simulink model "TosAeb"  (R2021a)
settings: Solver=FixedStepDiscrete  StartTime=0.0  StopTime=inf  FixedStep=0.01  RelTol=1e-3
undefined symbols (not defined in the file or in its folder): ACCModSts, AccSts1, AEB_ObjectMovingOrientation, AEB_ObjectMovingState, CN_BTCActive, CN_CurrentGearR, CN_CurrentGearR_DHT, CN_PTCActive, CN_VDCActive, CN_VSC_EspOff, CN_VSC_FCW_AEB_Error, CN_VSC_FCW_AEB_NoDispay, CN_VSC_FCW_AEB_PerfmDgdtn, CN_VSC_FCW_AEB_TempUnAvlbl, CN_VSC_VehStandstill, CNaccelAvoidMax, CNaccelAvoidMin, CNbrakePedalPress, CNcomfortStrLeftAccelMax, CNcomfortStrLeftAccelMin, CNcomfortStrRightAccelMax, CNcomfortStrRightAccelMin, CNDrvDoorOpen, CNDrvModeEco, CNDrvModeNorm, CNDrvModeOther, CNDrvModeSport, CNDrvSBRreminder, CNNull, CNobjInvalidID, CNPassDoorOpen, CNPassSBRreminder, CNPEBSAccelNegInf, CNPEBSAccelNull, CNPEBSTimeInf, CNPEBSTimeNull, CNSTOPMAX, CNSTOPNULL, CNsubjWidth, CNSwtOFF, CNSwtON, CNTTCMAX, CNTTCNULL, CNVehConfigAEBCycNone, CNVehConfigAEBNone, CNVehConfigAEBPedNone, CNVehConfigFCWNone, CNVehConfigJANone, CollisionSeverity, FALSE, FCWSnvty, JACycObjCalcInfoDefaultParams, JAPedObjCalcInfoDefaultParams, JAVehObjCalcInfoDefaultParams, latCycObjCalcInfoDefaultParams, latPedObjCalcInfoDefaultParams, longObjCalcInfoDefaultParams, ModSts, movingStatus, ObjectType ... (+1112)
libraries: simulink x3031, AEBSFunctionLib x50, Ta_Library x33, AEBSStateMachineLib x23, Mylibrary x12, simulink_extras x7, ObjSelect_lib x3, dspstat3 x2
(part 96 of 102)

== matlab function TosAeb/AEB_Objsel/TA_Processor/PreProcessor/ObjectPrecessor/TAObjInfoCalc/For Each Subsystem/Info_Check1/Type_Process/ObjType_rebuild ==
  data: Obj_ID input; Obj_type input; C_ObjType output; Obj_Length input; Obj_Width input; Obj_Height input; Obj_dx input; abs_Spd input; P_TA_ObjTruckLengthPara input; P_TA_ObjMotorVehSpdThd input; P_TA_ObjPedestrainSpdThd input; C_ObjLength output; C_ObjWidth output; C_ObjHight output; C_Objdx output
function [C_ObjType,C_ObjLength,C_ObjWidth,C_ObjHight,C_Objdx]  = fcn(Obj_ID,Obj_type,Obj_Length,Obj_Width,Obj_Height,Obj_dx,abs_Spd,P_TA_ObjTruckLengthPara,P_TA_ObjMotorVehSpdThd,P_TA_ObjPedestrainSpdThd)
% P_TA_ObjTruckWidthPara,P_TA_ObjCarLengthPara,P_TA_ObjCarWidthPara,...
% P_TA_ObjMotorBicycleLengthPara,P_TA_ObjMotorBicycleWidthPara,P_TA_ObjBicycleLengthPara,P_TA_ObjBicycleWidthPara,P_TA_ObjPedestrainLengthPara,...
% P_TA_ObjPedestrainWidthPara,P_TA_ObjBusLengthPara,P_TA_ObjBusWidthPara,P_TA_ObjLongthValidFactor,P_TA_ObjWidthValidFactor
%Fus_ObjType 传感器输出目标类型，对应参数如下：
% 0: unknown  1: Bus  2: Small_Medium_Car (中小型轿车) 3: Trucks (大卡车)  4: Motors (三轮车)
% 5: Special_vehicle (特殊车) 6: Tiny_car (微型车) 7: Lorry (小货车) 8: MiniVan (面包车)
% 9: Motor Cyclist(摩托车) 10：Cyclist（自行车）11：Pedestrian(Adult) 12:Pedestrian（child）
% 13: others(动物或不能划分清楚的)
%重新校验后目标类型如下：
%0x0: Unknwon 0x1: Bus 0x2: Car 0x3: Truck 0x4: Motor Bike
%0x5: Bicycle 0x6: Pedestrian 0x7: Animals
C_ObjType = uint8(0);
C_ObjLength = single(0);
C_ObjWidth = single(0);
C_ObjHight = single(0);
C_Objdx = single(0);
if Obj_ID==uint8(0)
    C_ObjType = uint8(0);
    C_ObjLength = single(0);
    C_ObjWidth = single(0);
    C_ObjHight = single(0);
    C_Objdx = single(0);
elseif Obj_type==uint8(13)
    if Obj_Length~=single(0)&&Obj_Width~=single(0)
        C_ObjType = uint8(0);
        C_ObjLength = Obj_Length;
        C_ObjWidth = Obj_Width;
        C_ObjHight = Obj_Height;
        C_Objdx = Obj_dx;
    else
        C_ObjType = uint8(0);
        C_ObjLength = single(0);
        C_ObjWidth = single(0);
        C_ObjHight = single(0);
        C_Objdx = Obj_dx;
    end
elseif Obj_type == uint8(1)||Obj_type == uint8(3)||Obj_type == uint8(5)||Obj_type == uint8(7)
    if Obj_type == uint8(1)
        C_ObjType = uint8(1);
        C_ObjLength = Obj_Length;
        C_ObjWidth = Obj_Width;
        C_ObjHight = Obj_Height;
        C_Objdx = Obj_dx;
    elseif Obj_type == uint8(3)||(Obj_type == uint8(5)&&Obj_Length>P_TA_ObjTruckLengthPara)
        C_ObjType = uint8(3);
        C_ObjLength = Obj_Length;
        C_ObjWidth = Obj_Width;
        C_ObjHight = Obj_Height;
        C_Objdx = Obj_dx;
    elseif Obj_type == uint8(7)||(Obj_type == uint8(5)&&Obj_Length<P_TA_ObjTruckLengthPara)
        C_ObjType = uint8(3);
        C_ObjLength = Obj_Length;
        C_ObjWidth = Obj_Width;
        C_ObjHight = Obj_Height;
        C_Objdx = Obj_dx;
    else
        C_ObjType = uint8(0);
        C_ObjLength = Obj_Length;
        C_ObjWidth = Obj_Width;
        C_ObjHight = Obj_Height;
        C_Objdx = Obj_dx;
    end
elseif Obj_type == uint8(2)||Obj_type == uint8(4)||Obj_type == uint8(6)||Obj_type == uint8(8)
    C_ObjType = uint8(2);
    C_ObjLength = Obj_Length;
    C_ObjWidth = Obj_Width;
    C_ObjHight = Obj_Height;
    C_Objdx = Obj_dx;
elseif Obj_type == uint8(9)||Obj_type == uint8(10)||Obj_type == uint8(11)||Obj_type == uint8(12)
    if (Obj_type == uint8(11)||Obj_type == uint8(12))&&abs_Spd>P_TA_ObjPedestrainSpdThd
        C_ObjType = uint8(5);
        C_ObjLength = Obj_Length;
        C_ObjWidth = Obj_Width;
        C_ObjHight = Obj_Height;
        C_Objdx = Obj_dx;
    elseif (Obj_type == uint8(11)||Obj_type == uint8(12))&&abs_Spd<P_TA_ObjPedestrainSpdThd
        C_ObjType = uint8(6);
        C_ObjLength = single(0.5);
        C_ObjWidth = single(0.5);
        C_ObjHight = Obj_Height;
        C_Objdx = Obj_dx;
    elseif Obj_type == uint8(9)  %||Obj_type == uint8(10)
        if abs_Spd>=P_TA_ObjMotorVehSpdThd
            C_ObjType = uint8(4);
            C_ObjLength = Obj_Length;
            C_ObjWidth = Obj_Width;
            C_ObjHight = Obj_Height;
            C_Objdx = Obj_dx;
        elseif abs_Spd<P_TA_ObjMotorVehSpdThd && abs_Spd>P_TA_ObjPedestrainSpdThd
            C_ObjType = uint8(5);
            C_ObjLength = Obj_Length;
            C_ObjWidth = Obj_Width;
            C_ObjHight = Obj_Height;
            C_Objdx = Obj_dx;
        else
            if Obj_Length >single(1)||Obj_Width > single(1)
                C_ObjType = uint8(4);
                C_ObjLength = Obj_Length;
                C_ObjWidth = Obj_Width;
                C_ObjHight = Obj_Height;
                C_Objdx = Obj_dx;
            else
                C_ObjType = uint8(6);
                C_ObjLength = single(0.5);
                C_ObjWidth = single(0.5);
                C_ObjHight = Obj_Height;
                C_Objdx = Obj_dx;
            end
        end
    elseif Obj_type == uint8(10)
        C_ObjType = uint8(5);
        C_ObjLength = Obj_Length;
        C_ObjWidth = Obj_Width;
        C_ObjHight = Obj_Height;
        C_Objdx = Obj_dx;
    end
else
    C_ObjType = uint8(0);
    C_ObjLength = Obj_Length;
    C_ObjWidth = Obj_Width;
    C_ObjHight = Obj_Height;
    C_Objdx = Obj_dx;
end
end
% Bound_Vecter = single(zeros(1,6));
% %目标存在时
% if Obj_type ~= uint8(0)
%     Bound_Vecter(1) = vecter_cal(Obj_Length,Obj_Width,P_TA_ObjTruckLengthPara,P_TA_ObjTruckWidthPara);
%     Bound_Vecter(2) = vecter_cal(Obj_Length,Obj_Width,P_TA_ObjCarLengthPara,P_TA_ObjCarWidthPara);
%     Bound_Vecter(3) = vecter_cal(Obj_Length,Obj_Width,P_TA_ObjBusLengthPara,P_TA_ObjBusWidthPara);
%     Bound_Vecter(4) = vecter_cal(Obj_Length,Obj_Width,P_TA_ObjMotorBicycleLengthPara,P_TA_ObjMotorBicycleWidthPara);
%     Bound_Vecter(5) = vecter_cal(Obj_Length,Obj_Width,P_TA_ObjBicycleLengthPara,P_TA_ObjBicycleWidthPara);
%     Bound_Vecter(6) = vecter_cal(Obj_Length,Obj_Width,P_TA_ObjPedestrainLengthPara,P_TA_ObjPedestrainWidthPara);
%     [~,I] = sort(Bound_Vecter);
%     if Obj_type == uint8(3)
%         %Truck相关数据处理
%         if I(1) == uint8(1)
%             C_ObjType = uint8(3);
%             C_ObjLength = Obj_Length;
%             C_ObjWidth = Obj_Width;
%             C_ObjHight = Obj_Height;
%             C_Objdx = Obj_dx;
%         else
%             [t_flg] = caul(Obj_Length,Obj_Width,P_TA_ObjTruckLengthPara,P_TA_ObjTruckWidthPara,P_TA_ObjLongthValidFactor,P_TA_ObjWidthValidFactor);
%             C_Objdx = Obj_dx;
%             if t_flg == true && Obj_Height < single(1.9)
%                 if abs_Spd >= P_TA_ObjMotorVehSpdThd
%                     C_ObjType = uint8(2);
%                     C_ObjLength = P_TA_ObjCarLengthPara;
%                     C_ObjWidth = P_TA_ObjCarWidthPara;
%                     C_ObjHight = single(1.85);
%                 elseif abs_Spd < P_TA_ObjMotorVehSpdThd && abs_Spd >= P_TA_ObjPedestrainSpdThd
%                     C_ObjType = uint8(5);
%                     C_ObjLength = P_TA_ObjBicycleLengthPara;
%                     C_ObjWidth = P_TA_ObjBicycleWidthPara;
%                     C_ObjHight = single(1.80);
%                 else
%                     C_ObjType = uint8(6);
%                     C_ObjLength = P_TA_ObjPedestrainLengthPara;
%                     C_ObjWidth = P_TA_ObjPedestrainWidthPara;
%                     C_ObjHight = single(1.75);
%                 end
%             else
%                 C_ObjType = uint8(0);
%                 C_ObjLength = Obj_Length;
%                 C_ObjWidth = Obj_Width;
%                 C_ObjHight = Obj_Height;
%             end
%         end
%     elseif Obj_type == uint8(2) || (Obj_type >= uint8(4) && Obj_type <= uint8(8))
%         %Car相关数据处理
%         if I(1) == uint8(2)
%             C_ObjType = uint8(2);
%             C_ObjLength = Obj_Length;
%             C_ObjWidth = Obj_Width;
%             C_ObjHight = Obj_Height;
%         else
%             [t_flg] = caul(Obj_Length,Obj_Width,P_TA_ObjCarLengthPara,P_TA_ObjCarWidthPara,P_TA_ObjLongthValidFactor,P_TA_ObjWidthValidFactor);
%             C_Objdx = Obj_dx;
%             if t_flg == true
%                 if abs_Spd >= P_TA_ObjMotorVehSpdThd
%                     C_ObjType = uint8(2);
%                     C_ObjLength = P_TA_ObjCarLengthPara;
%                     C_ObjWidth = P_TA_ObjCarWidthPara;
%                     C_ObjHight = single(1.85);
%                 elseif abs_Spd < P_TA_ObjMotorVehSpdThd && abs_Spd >= P_TA_ObjPedestrainSpdThd
%                     C_ObjType = uint8(5);
%                     C_ObjLength = P_TA_ObjBicycleLengthPara;
%                     C_ObjWidth = P_TA_ObjBicycleWidthPara;
%                     C_ObjHight = single(1.80);
%                 else
%                     C_ObjType = uint8(6);
%                     C_ObjLength = P_TA_ObjPedestrainLengthPara;
%                     C_ObjWidth = P_TA_ObjPedestrainWidthPara;
%                     C_ObjHight = single(1.75);
%                 end
%             else
%                 C_ObjType = uint8(0);
%                 C_ObjLength = Obj_Length;
%                 C_ObjWidth = Obj_Width;
%                 C_ObjHight = Obj_Height;
%             end
%         end
%         elseif Obj_type == uint8(1)
%             %bus相关数据处理
%             if I(1) == uint8(3)
%                 C_ObjType = uint8(1);
%                 C_ObjLength = Obj_Length;
%                 C_ObjWidth = Obj_Width;
%                 C_ObjHight = Obj_Height;
%             else
%                 [t_flg] = caul(Obj_Length,Obj_Width,P_TA_ObjBusLengthPara,P_TA_ObjBusWidthPara,P_TA_ObjLongthValidFactor,P_TA_ObjWidthValidFactor);
%                 C_Objdx = Obj_dx;
%                 if t_flg == true
%                     if abs_Spd >= P_TA_ObjMotorVehSpdThd
%                         C_ObjType = uint8(2);
%                         C_ObjLength = P_TA_ObjCarLengthPara;
%                         C_ObjWidth = P_TA_ObjCarWidthPara;
%                         C_ObjHight = single(1.85);
%                     elseif abs_Spd < P_TA_ObjMotorVehSpdThd && abs_Spd >= P_TA_ObjPedestrainSpdThd
%                         C_ObjType = uint8(5);
%                         C_ObjLength = P_TA_ObjBicycleLengthPara;
%                         C_ObjWidth = P_TA_ObjBicycleWidthPara;
%                         C_ObjHight = single(1.80);
%                     else
%                         C_ObjType = uint8(6);
%                         C_ObjLength = P_TA_ObjPedestrainLengthPara;
%                         C_ObjWidth = P_TA_ObjPedestrainWidthPara;
%                         C_ObjHight = single(1.75);
%                     end
%                 else
%                     C_ObjType = uint8(0);
%                     C_ObjLength = Obj_Length;
%                     C_ObjWidth = Obj_Width;
%                     C_ObjHight = Obj_Height;
%                 end
%             end
%     elseif Obj_type == uint8(9)
%         %Motor相关数据处理
%         if I(1) == uint8(4)
%             C_ObjType = uint8(4);
%             C_ObjLength = Obj_Length;
%             C_ObjWidth = Obj_Width;
%             C_ObjHight = Obj_Height;
%         else
%             [t_flg] = caul(Obj_Length,Obj_Width,P_TA_ObjMotorBicycleLengthPara,P_TA_ObjMotorBicycleWidthPara,P_TA_ObjLongthValidFactor,P_TA_ObjWidthValidFactor);
%             C_Objdx = Obj_dx;
%             if t_flg == true
%                 if abs_Spd >= P_TA_ObjMotorVehSpdThd
%                     C_ObjType = uint8(2);
%                     C_ObjLength = P_TA_ObjCarLengthPara;
%                     C_ObjWidth = P_TA_ObjCarWidthPara;
%                     C_ObjHight = single(1.85);
%                 elseif abs_Spd < P_TA_ObjMotorVehSpdThd && abs_Spd >= P_TA_ObjPedestrainSpdThd
%                     C_ObjType = uint8(5);
%                     C_ObjLength = P_TA_ObjBicycleLengthPara;
%                     C_ObjWidth = P_TA_ObjBicycleWidthPara;
%                     C_ObjHight = single(1.80);
%                 else
%                     C_ObjType = uint8(6);
%                     C_ObjLength = P_TA_ObjPedestrainLengthPara;
%                     C_ObjWidth = P_TA_ObjPedestrainWidthPara;
%                     C_ObjHight = single(1.75);
%                 end
%             else
%                 C_ObjType = uint8(0);
%                 C_ObjLength = Obj_Length;
%                 C_ObjWidth = Obj_Width;
%                 C_ObjHight = Obj_Height;
%             end
%         end
%     elseif Obj_type == uint8(10)
%         %Bicycle相关数据处理
%         if I(1) == uint8(5)||(Obj_Length == single(0)&& Obj_Width == single(0) && abs(Obj_Height) ~= single(0))
%             C_ObjType = uint8(5);
%             C_ObjLength = P_TA_ObjBicycleLengthPara;
%             C_ObjWidth = P_TA_ObjBicycleWidthPara;
%              C_ObjHight = single(1.80);
%         else
%             [t_flg] = caul(Obj_Length,Obj_Width,P_TA_ObjBicycleLengthPara,P_TA_ObjBicycleWidthPara,P_TA_ObjLongthValidFactor,P_TA_ObjWidthValidFactor);
%             C_Objdx = Obj_dx;
%             if t_flg == true
%                 if abs_Spd >= P_TA_ObjMotorVehSpdThd
%                     C_ObjType = uint8(2);
%                     C_ObjLength = P_TA_ObjCarLengthPara;
%                     C_ObjWidth = P_TA_ObjCarWidthPara;
%                     C_ObjHight = single(1.85);
%                 elseif abs_Spd < P_TA_ObjMotorVehSpdThd && abs_Spd >= P_TA_ObjPedestrainSpdThd
%                     C_ObjType = uint8(5);
%                     C_ObjLength = P_TA_ObjBicycleLengthPara;
%                     C_ObjWidth = P_TA_ObjBicycleWidthPara;
%                     C_ObjHight = single(1.80);
%                 else
%                     C_ObjType = uint8(6);
%                     C_ObjLength = P_TA_ObjPedestrainLengthPara;
%                     C_ObjWidth = P_TA_ObjPedestrainWidthPara;
%                     C_ObjHight = single(1.75);
%                 end
%             else
%                 C_ObjType = uint8(0);
%                 C_ObjLength = Obj_Length;
%                 C_ObjWidth = Obj_Width;
%                 C_ObjHight = Obj_Height;
%             end
%         end
%     elseif Obj_type == uint8(11) || Obj_type == uint8(12)
%         %Pedestrian相关数据处理
%         if I(1) == uint8(6)||(Obj_Length == single(0)&& Obj_Width == single(0) && abs(Obj_Height) ~= single(0))
%             C_ObjType = uint8(6);
%             C_ObjLength = P_TA_ObjPedestrainLengthPara;
%             C_ObjWidth = P_TA_ObjPedestrainWidthPara;
%             C_ObjHight = Obj_Height;
%         else
%             [t_flg] = caul(Obj_Length,Obj_Width,P_TA_ObjPedestrainLengthPara,P_TA_ObjPedestrainWidthPara,P_TA_ObjLongthValidFactor,P_TA_ObjWidthValidFactor);
%             C_Objdx = Obj_dx;
%             if t_flg == true
%                 if abs_Spd >= P_TA_ObjMotorVehSpdThd
%                     C_ObjType = uint8(2);
%                     C_ObjLength = P_TA_ObjCarLengthPara;
%                     C_ObjWidth = P_TA_ObjCarWidthPara;
%                     C_ObjHight = single(1.85);
%                 elseif abs_Spd < P_TA_ObjMotorVehSpdThd && abs_Spd >= P_TA_ObjPedestrainSpdThd
%                     C_ObjType = uint8(5);
%                     C_ObjLength = P_TA_ObjBicycleLengthPara;
%                     C_ObjWidth = P_TA_ObjBicycleWidthPara;
%                     C_ObjHight = single(1.80);
%                 else
%                     C_ObjType = uint8(6);
%                     C_ObjLength = P_TA_ObjPedestrainLengthPara;
%                     C_ObjWidth = P_TA_ObjPedestrainWidthPara;
%                     C_ObjHight = single(1.75);
%                 end
%             else
%                 C_ObjType = uint8(0);
%                 C_ObjLength = Obj_Length;
%                 C_ObjWidth = Obj_Width;
%                 C_ObjHight = Obj_Height;
%             end
%         end
%     else
%         C_ObjType = uint8(7);
%         C_ObjLength = Obj_Length;
%         C_ObjWidth = Obj_Width;
%         C_ObjHight = Obj_Height;
%     end
% end
% end
% %% 初步判断
% function factor = vecter_cal(Obj_longth,Obj_Widths,PA_long,PA_width)
% f_long = (Obj_longth - PA_long)/Obj_longth>0;
% f_width = (Obj_Widths - PA_width)/Obj_Widths>0;
% factor = f_long * f_width;
% end
% %% 对目标长宽进行校核
% function [t_ChangeFlg] = caul(Obj_long,Obj_Width,P_long,P_width,P_longFactor,P_WidthFactor)
% if Obj_long < single(0) || Obj_Width < single(0)
%     t_ChangeFlg = true;
% elseif  abs(Obj_long - P_long) > P_longFactor * Obj_long && abs(Obj_Width - P_width) > P_WidthFactor * Obj_Width
%     t_ChangeFlg = true;
% else
%     t_ChangeFlg = false;
% end
% end

== matlab function TosAeb/AEB_Objsel/TA_Processor/PreProcessor/VehiclePreProcessor/CalculateCurvature/SafeDivide/SafeDivide ==
  data: Numerator input; Quotient output; Denominator input; DataTypeMin parameter; DataTypeMax parameter
function Quotient = fcn(Numerator, Denominator, DataTypeMin, DataTypeMax)
    %#codegen
    
    % Test for division by zero and overflow
    if abs(Denominator) > abs(Numerator)*(1/DataTypeMax)
        % Normal divide
        Quotient=Numerator/Denominator;
    else
        % Select output depending on the numerator
        if Numerator>0
            % x/0
            Quotient=DataTypeMax;
        elseif Numerator<0
            % -x/0
            Quotient=DataTypeMin;
        else
            % 0/0
            Quotient=cast(0,class(Numerator));
        end
    end
end

== matlab function TosAeb/AEB_Objsel/TA_Processor/PreProcessor/VehiclePreProcessor/CalculateCurvature/SafeDivide1/SafeDivide ==
  data: Numerator input; Quotient output; Denominator input; DataTypeMin parameter; DataTypeMax parameter
function Quotient = fcn(Numerator, Denominator, DataTypeMin, DataTypeMax)
    %#codegen
    
    % Test for division by zero and overflow
    if abs(Denominator) > abs(Numerator)*(1/DataTypeMax)
        % Normal divide
        Quotient=Numerator/Denominator;
    else
        % Select output depending on the numerator
        if Numerator>0
            % x/0
            Quotient=DataTypeMax;
        elseif Numerator<0
            % -x/0
            Quotient=DataTypeMin;
        else
            % 0/0
            Quotient=cast(0,class(Numerator));
        end
    end
end

== matlab function TosAeb/AEB_Objsel/TA_Processor/PreProcessor/VehiclePreProcessor/CalculateCurvature/StaticBicycleModelC0/CalculateWheelAngle/SafeDivide/SafeDivide ==
  data: Numerator input; Quotient output; Denominator input; DataTypeMin parameter; DataTypeMax parameter
function Quotient = fcn(Numerator, Denominator, DataTypeMin, DataTypeMax)
    %#codegen
    
    % Test for division by zero and overflow
    if abs(Denominator) > abs(Numerator)*(1/DataTypeMax)
        % Normal divide
        Quotient=Numerator/Denominator;
    else
        % Select output depending on the numerator
        if Numerator>0
            % x/0
            Quotient=DataTypeMax;
        elseif Numerator<0
            % -x/0
            Quotient=DataTypeMin;
        else
            % 0/0
            Quotient=cast(0,class(Numerator));
        end
    end
end

== matlab function TosAeb/AEB_Objsel/TA_Processor/PreProcessor/VehiclePreProcessor/CalculateCurvature/StaticBicycleModelC0/IntermediateCalculation/SafeDivide/SafeDivide ==
  data: Numerator input; Quotient output; Denominator input; DataTypeMin parameter; DataTypeMax parameter
function Quotient = fcn(Numerator, Denominator, DataTypeMin, DataTypeMax)
    %#codegen
    
    % Test for division by zero and overflow
    if abs(Denominator) > abs(Numerator)*(1/DataTypeMax)
        % Normal divide
        Quotient=Numerator/Denominator;
    else
        % Select output depending on the numerator
        if Numerator>0
            % x/0
            Quotient=DataTypeMax;
        elseif Numerator<0
            % -x/0
            Quotient=DataTypeMin;
        else
            % 0/0
            Quotient=cast(0,class(Numerator));
        end
    end
end

== matlab function TosAeb/AEB_Objsel/TA_Processor/PreProcessor/VehiclePreProcessor/CalculateCurvature/StaticBicycleModelC1/CalculateWheelAngle/SafeDivide/SafeDivide ==
  data: Numerator input; Quotient output; Denominator input; DataTypeMin parameter; DataTypeMax parameter
function Quotient = fcn(Numerator, Denominator, DataTypeMin, DataTypeMax)
    %#codegen
    
    % Test for division by zero and overflow
    if abs(Denominator) > abs(Numerator)*(1/DataTypeMax)
        % Normal divide
        Quotient=Numerator/Denominator;
    else
        % Select output depending on the numerator
        if Numerator>0
            % x/0
            Quotient=DataTypeMax;
        elseif Numerator<0
            % -x/0
            Quotient=DataTypeMin;
        else
            % 0/0
            Quotient=cast(0,class(Numerator));
        end
    end
end

== matlab function TosAeb/AEB_Objsel/TA_Processor/AEB_ObjSelect/TargetSelect/Long_ObjSelect/Obj_IDConfirm/Target_Select_decle/Obj_ConfirmFunc ==
  data: re_Valid output; Obj_ID input; Obj_vx input; Obj_state input; Obj_Orentation input; Obj_dy input; Obj_dx input; obj_vxabs input; Subj_vx input; Obj_Coef input; TTI input; Overlap_Rate input; P_TA_PrmForVehSpdStationary input; P_TA_PrmForObjSpdStationary input; re_ID output; re_dx output; re_dy output; re_TTC output
function [re_Valid,re_ID,re_dx,re_dy,re_TTC] = fcn(Obj_ID,Obj_vx,Obj_state,Obj_Orentation,Obj_dy,Obj_dx,obj_vxabs,Subj_vx,Obj_Coef,TTI,Overlap_Rate,P_TA_PrmForVehSpdStationary,P_TA_PrmForObjSpdStationary)
t_Obj_Valid  = uint8((zeros(32,1)));
t_Obj_ID = uint8((zeros(32,1)));
t_Obj_dy = single(200)*single(ones(32,1));
t_Obj_dx = single(200)*single(ones(32,1));
t_TTI = single(100)*single(ones(32,1));
% 判断自车运动状态
if Subj_vx <= P_TA_PrmForVehSpdStationary
    t_Obj_Valid = uint8((zeros(32,1)));
    t_Obj_ID = uint8((zeros(32,1)));
    t_Obj_dy = single(200)*single(ones(32,1));
    t_Obj_dx = single(200)*single(ones(32,1));
    t_TTI = single(100)*single(ones(32,1));
else
    for i = 1:32
        %判断目标的有效性
        %目标运动状态未知、当前及预测状态下均不存在风险及不符合逻辑的静止目标
        if Obj_Coef(i) == uint8(255) || Obj_Coef(i) == uint8(0) || Obj_state(i) == uint8(0) ||...
                ((Obj_Orentation(i) == uint8(0)||(Obj_vx(i) + Subj_vx <= P_TA_PrmForObjSpdStationary || obj_vxabs(i)<P_TA_PrmForObjSpdStationary))&&Overlap_Rate(i) == false) %静止目标筛除
            t_Obj_Valid(i) = uint8(0);
            t_Obj_ID(i) = uint8(0);
            t_Obj_dy(i) = single(200);
            t_Obj_dx(i) = single(200);
            t_TTI(i) = single(100);
        else
            t_Obj_Valid(i) = uint8(1);
            t_Obj_ID(i) = Obj_ID(i);
            t_Obj_dy(i) = Obj_dy(i);
            t_Obj_dx(i) = Obj_dx(i);
            t_TTI(i) = TTI(i);
        end
    end
end
re_Valid = t_Obj_Valid;
re_ID = t_Obj_ID;
re_dx = t_Obj_dx;
re_dy = t_Obj_dy;
re_TTC = t_TTI;
end

== matlab function TosAeb/AEB_Objsel/TA_Processor/TA_Check/TA_LatCheck/Lat_Check ==
  data: Lat_ObjID input; ID output; Lat_ObjSortNum input; Lat_TTC input; Num output; Ttc output
function [ID,Num,Ttc] = fcn(Lat_ObjID,Lat_ObjSortNum,Lat_TTC)
t_ID = uint8(zeros(10,1));
t_Num = uint8(zeros(10,1));
t_Ttc=single(zeros(10,1));
for i = 1:10
    if Lat_ObjID(i) == uint8(0)
        t_ID(i) = uint8(0);
        t_Num(i) = uint8(255);
        t_Ttc(i) = single(100);
    else
        t_ID(i) = Lat_ObjID(i);
        t_Num(i) = Lat_ObjSortNum(i);
        t_Ttc(i) = Lat_TTC(i);
    end     
end
ID = t_ID;
Num = t_Num;
Ttc = t_Ttc;
end

== matlab function TosAeb/AEB_Objsel/TA_Processor/TA_Check/TA_LongCheck/ID_Confirm ==
  data: ID output; ObjSortNum output; Obs_ID input; TTC output; CIPV_ID input; MPC_ID input; CIPV_TtcValid input; CIPV_Ttc input; MPC_TtcValid input; MPC_Ttc input; Long_ObjID input; Long_TTC input; Long_ObjSortNum input
function [ID,ObjSortNum,TTC] = fcn(Obs_ID,CIPV_ID,MPC_ID,CIPV_TtcValid,CIPV_Ttc,MPC_TtcValid,MPC_Ttc,Long_ObjID,Long_TTC,Long_ObjSortNum)
 
if CIPV_ID == uint8(0) && MPC_ID == uint8(0) && CIPV_TtcValid == false && MPC_TtcValid == false
    Change_Flg = uint8(0);
    ID = Long_ObjID;
    TTC = Long_TTC(Long_ObjSortNum);
elseif (CIPV_ID ~= uint8(0) && CIPV_TtcValid == true) && (MPC_ID == uint8(0) && MPC_TtcValid == false)
    if CIPV_ID == Long_ObjID
        Change_Flg = uint8(0);
        ID = Long_ObjID;
        TTC = Long_TTC(Long_ObjSortNum);
    else
        if CIPV_Ttc >= Long_TTC(Long_ObjSortNum)
            Change_Flg = uint8(0);
            ID = Long_ObjID;
            TTC = Long_TTC(Long_ObjSortNum);
        else
            Change_Flg = uint8(1);
            ID = CIPV_ID;
            TTC = CIPV_Ttc;
        end
    end
elseif (CIPV_ID == uint8(0) && CIPV_TtcValid == false) && (MPC_ID ~= uint8(0) && MPC_TtcValid ==true)
    if MPC_ID == Long_ObjID
        Change_Flg = uint8(0);
        ID = Long_ObjID;
        TTC = Long_TTC(Long_ObjSortNum);
    else
        if MPC_Ttc >= Long_TTC(Long_ObjSortNum)
            Change_Flg = uint8(0);
            ID = Long_ObjID;
            TTC = Long_TTC(Long_ObjSortNum);
        else
            Change_Flg = uint8(1);
            ID = MPC_ID;
            TTC = MPC_Ttc;
        end
    end
else
    if (CIPV_ID ~= uint8(0) && CIPV_TtcValid == true) && (MPC_ID ~= uint8(0) && MPC_TtcValid == true)
        if CIPV_Ttc<= MPC_Ttc
            if CIPV_ID == Long_ObjID
                Change_Flg = uint8(0);
                ID = Long_ObjID;
                TTC = Long_TTC(Long_ObjSortNum);
            else
                if CIPV_Ttc >= Long_TTC(Long_ObjSortNum)
                    Change_Flg = uint8(0);
                    ID = Long_ObjID;
                    TTC = Long_TTC(Long_ObjSortNum);
                else
                    Change_Flg = uint8(1);
                    ID = CIPV_ID;
                    TTC = CIPV_Ttc;
                end
            end
        else
            if MPC_ID == Long_ObjID
                Change_Flg = uint8(0);
                ID = Long_ObjID;
                TTC = Long_TTC(Long_ObjSortNum);
            else
                if MPC_Ttc >= Long_TTC(Long_ObjSortNum)
                    Change_Flg = uint8(0);
                    ID = Long_ObjID;
                    TTC = Long_TTC(Long_ObjSortNum);
                else
                    Change_Flg = uint8(1);
                    ID = MPC_ID;
                    TTC = MPC_Ttc;
                end
            end
        end
    else
        Change_Flg = uint8(0);
        ID = Long_ObjID;
        TTC = Long_TTC(Long_ObjSortNum);
    end
end
if Change_Flg == uint8(0)
    ObjSortNum = Long_ObjSortNum;
else

     if Obs_ID == ID
         ObjSortNum = Long_ObjSortNum;
     else
         ObjSortNum = uint8(0);
    end
end

== stateflow chart TosAeb/ELK_Objsel/ELK_Left_Obj_Delay/Hysteresis2 ==
  state Flag_False
    en:
    Flag = 0;
    du:
    Flag = 0;
    settings: description=_GWMH9P5.ELKFN.111_3_
  state Flag_True
    en:
    Flag = 1;
    du:
    Flag = 1;
    settings: description=_GWMH9P5.ELKFN.111_4_
  (default) -> Flag_False
  Flag_False -> Flag_True : [Input_1 == 1]
  Flag_True -> Flag_False : [Input_2 == 1]
  data: Input_1 input; Input_2 input; Flag output double

== stateflow chart TosAeb/ELK_Objsel/ELK_Left_Obj_Delay/Trigger Hold1/Chart ==
  (default) -> (junction 1)
  (junction 1) -> (junction 2) [order 1] : [(Trigger==1)]...
      | {count = 0;}
  (junction 1) -> (junction 12) [order 2]
  (junction 2) -> (junction 10)
  (junction 12) -> (junction 10) [order 1] : [(count<= hold_time)&&(count>0)]
  (junction 12) -> (junction 3) [order 2] : {Out = 0;}
  (junction 10) -> (junction 15) : {
      | Out = 1;...
      | count = count + 10;
      | }
  (junction 3) -> (junction 15)
  (junction 15) -> (junction 8)
  data: Trigger input; hold_time input; Out output double; count local double

== stateflow chart TosAeb/FcwAebJa_Step_sys/ALG_AEBSystem/ALG_AEBController/AEB_FuncCondCalcApp/CycFunctionCondCalc/cycAEBfullSuppAborCond/cycAEBfullAborCondNoStandstillCalc/cycAEBfullObjRelActiveCondCalc/Chart ==
  (default) -> (junction 2)
  (junction 2) -> (junction 3) [order 1] : [targetObjID ~= CNobjInvalidID]
  (junction 2) -> (junction 26) [order 2] : {
      | 	cycAEBfullObjRelAborCondFulfilled = true;
      | }
  (junction 3) -> (junction 16)
  (junction 16) -> (junction 18) [order 1] : [targetObjID == targetObjIDObjCalcK1]
  (junction 16) -> (junction 20) [order 2] : {
      | 	cycAEBfullObjRelAborCondFulfilled = true;
      | }
  (junction 18) -> (junction 5)
  (junction 5) -> (junction 7) [order 1] : [objIsFusionTarget == true]
  (junction 5) -> (junction 11) [order 2]
  (junction 7) -> (junction 9) : {
      | 	cycAEBfullObjRelAborCondFulfilled = false;
      | }
  (junction 11) -> (junction 14) [order 1] : [objLatCollisionProb <= P_cycAEBfulColliisonRiskProbAborThresObjID]
  (junction 11) -> (junction 24) [order 2] : {
      | 	cycAEBfullObjRelAborCondFulfilled = false;
      | }
  (junction 14) -> (junction 22) : {
      | 	cycAEBfullObjRelAborCondFulfilled = true;
      | }
  (junction 20) -> (junction 26)
  (junction 24) -> (junction 20)
  (junction 22) -> (junction 24)
  (junction 9) -> (junction 22)
  (junction 26) -> (junction 32)
  data: targetObjID input; targetObjIDObjCalcK1 input; objIsFusionTarget input; objLatCollisionProb input; cycAEBfullObjRelAborCondFulfilled output boolean; CNobjInvalidID parameter; P_cycAEBfulColliisonRiskProbAborThresObjID input

== stateflow chart TosAeb/FcwAebJa_Step_sys/ALG_AEBSystem/ALG_AEBController/AEB_FuncCondCalcApp/CycFunctionCondCalc/cycAEBpartSuppAborCond/cycAEBpartAborCondNoStandstillCalc/cycAEBpartObjRelActiveCondCalc/cycAEBpartObjRelAborCondFlow ==
  (default) -> (junction 2)
  (junction 2) -> (junction 3) [order 1] : [targetObjID ~= CNobjInvalidID]
  (junction 2) -> (junction 26) [order 2] : {
      | 	cycAEBfullObjRelAborCondFulfilled = true;
      | }
  (junction 3) -> (junction 16)
  (junction 16) -> (junction 18) [order 1] : [targetObjID == targetObjIDObjCalcK1]
  (junction 16) -> (junction 20) [order 2] : {
      | 	cycAEBfullObjRelAborCondFulfilled = true;
      | }
  (junction 18) -> (junction 5)
  (junction 5) -> (junction 7) [order 1] : [objIsFusionTarget == true]
  (junction 5) -> (junction 11) [order 2]
  (junction 7) -> (junction 9) : {
      | 	cycAEBfullObjRelAborCondFulfilled = false;
      | }
  (junction 11) -> (junction 14) [order 1] : [objLatCollisionProb <= P_cycAEBpartColliisonRiskProbAborThresObjID]
  (junction 11) -> (junction 24) [order 2] : {
      | 	cycAEBfullObjRelAborCondFulfilled = false;
      | }
  (junction 14) -> (junction 22) : {
      | 	cycAEBfullObjRelAborCondFulfilled = true;
      | }
  (junction 20) -> (junction 26)
  (junction 24) -> (junction 20)
  (junction 22) -> (junction 24)
  (junction 9) -> (junction 22)
  (junction 26) -> (junction 32)
  data: targetObjID input; targetObjIDObjCalcK1 input; objIsFusionTarget input; objLatCollisionProb input; cycAEBfullObjRelAborCondFulfilled output boolean; CNobjInvalidID parameter; P_cycAEBpartColliisonRiskProbAborThresObjID input

== stateflow chart TosAeb/ELK_Objsel/ELK_Left_Obj_Delay1/Hysteresis2 ==
  state Flag_False
    en:
    Flag = 0;
    du:
    Flag = 0;
    settings: description=_GWMH9P5.ELKFN.111_3_
  state Flag_True
    en:
    Flag = 1;
    du:
    Flag = 1;
    settings: description=_GWMH9P5.ELKFN.111_4_
  (default) -> Flag_False
  Flag_False -> Flag_True : [Input_1 == 1]
  Flag_True -> Flag_False : [Input_2 == 1]
  data: Input_1 input; Input_2 input; Flag output double

== stateflow chart TosAeb/ELK_Objsel/ELK_Left_Obj_Delay1/Trigger Hold1/Chart ==
  (default) -> (junction 1)
  (junction 1) -> (junction 2) [order 1] : [(Trigger==1)]...
      | {count = 0;}
  (junction 1) -> (junction 12) [order 2]
  (junction 2) -> (junction 10)
  (junction 12) -> (junction 10) [order 1] : [(count<= hold_time)&&(count>0)]
  (junction 12) -> (junction 3) [order 2] : {Out = 0;}
  (junction 10) -> (junction 15) : {
      | Out = 1;...
      | count = count + 10;
      | }
  (junction 3) -> (junction 15)
  (junction 15) -> (junction 8)
  data: Trigger input; hold_time input; Out output double; count local double

== matlab function TosAeb/ELK_Objsel/LanesSelect/LineInfo_Process/Line_Info ==
  data: seg_num input; seg1_valid input; Valid output; seg1_Start input; seg1_end input; seg1_C0 input; seg1_C1 input; seg1_C2 input; seg1_C3 input; seg2_valid input; seg2_Start input; seg2_end input; seg2_C0 input; seg2_C1 input; seg2_C2 input; seg2_C3 input; seg3_valid input; seg3_Start input; seg3_end input; seg3_C0 input; seg3_C1 input; seg3_C2 input; seg3_C3 input; Line_start output; Line_end output; C0 output; C1 output; C2 output; C3 output
function [Valid,Line_start,Line_end,C0,C1,C2,C3] = fcn(seg_num,seg1_valid,seg1_Start,seg1_end,seg1_C0,seg1_C1,seg1_C2,seg1_C3,...
    seg2_valid,seg2_Start,seg2_end,seg2_C0,seg2_C1,seg2_C2,seg2_C3,...
    seg3_valid,seg3_Start,seg3_end,seg3_C0,seg3_C1,seg3_C2,seg3_C3)
posx = single(zeros(20,1));
posy = single(zeros(20,1));
Valid = false;
Line_start = single(0);
Line_end = single(0);
C0 = single(0);
C1 = single(0);
C2 = single(0);
C3 = single(0);
%确定有效分段数目
if seg_num == uint8(0)

    %只有一段距离有效，输出有效段参数，后续需要增加距离判断拟合
elseif seg_num == uint8(1)
    if seg1_valid == true && seg2_valid ==false && seg3_valid == false
        [Valid,Line_start,Line_end,C0,C1,C2,C3] = func_assign(seg1_valid,seg1_Start,seg1_end,seg1_C0,seg1_C1,seg1_C2,seg1_C3);
    elseif seg2_valid == true && seg1_valid ==false && seg3_valid == false
        [Valid,Line_start,Line_end,C0,C1,C2,C3] = func_assign(seg2_valid,seg2_Start,seg2_end,seg2_C0,seg2_C1,seg2_C2,seg2_C3);
    elseif seg3_valid == true && seg1_valid ==false && seg2_valid == false
        [Valid,Line_start,Line_end,C0,C1,C2,C3] = func_assign(seg3_valid,seg3_Start,seg3_end,seg3_C0,seg3_C1,seg3_C2,seg3_C3);
    else

    end
    %存在两端距离有效，判断是1、2，2、3，或者1、3（当前未考虑C0拼接的问题，需根据后续实车数据查看偏差对功能影响程度）
elseif seg_num == uint8(2)
    %1、2段距离有效
    if seg1_valid == true && seg2_valid == true && seg3_valid == false
        if seg1_Start > seg2_Start     %1、2段存在重叠
            if seg2_Start > single(0)  %1段的有效距离大于零
                seg2_Start = seg1_Start;
            else
                %seg1_Start = seg2_Start;
            end
        else
            seg2_Start  = seg1_Start;
        end
        t_size = func_step(seg1_end,seg2_end);
        for i = 1:20
            t_dx = seg1_end + (i - 1)*t_size;
            posx(i) = t_dx;
            if t_dx <= seg2_Start
                posy(i) = func_takePoint(seg1_C0,seg1_C1,seg1_C2,seg1_C3,t_dx);
            else
                posy(i) = func_takePoint(seg2_C0,seg2_C1,seg2_C2,seg2_C3,t_dx);
            end
        end
        coef = polyfit(posx,posy,3);
        Valid = true;
        Line_start = seg1_end;
        Line_end = seg2_end;
        C0 = coef(4);
        C1 = coef(3);
        C2 = coef(2);
        C3 = coef(1);
        %2、3段距离有效
    elseif seg1_valid == false && seg2_valid == true && seg3_valid == true
        if seg2_Start > single(30)       %(此处阈值的设置最好可以根据车速进行插值设置，后续需要根据实车数据进行处理)
            Valid = false;
            Line_start = single(0);
            Line_end = single(0);
            C0 = single(0);
            C1 = single(0);
            C2 = single(0);
            C3 = single(0);
        else
            if seg2_end < seg3_end
                if seg2_end <= seg3_Start     %2、3段存在重叠
                    seg3_Start = seg2_end;
                end
            else
                seg3_end = seg2_end;
            end
            t_size = func_step(seg2_Start,seg3_end);
            for i = 1:20
                t_dx = seg2_Start + (i - 1)*t_size;
                posx(i) = t_dx;
                if t_dx <= seg3_Start
                    posy(i) = func_takePoint(seg2_C0,seg2_C1,seg2_C2,seg2_C3,t_dx);
                else
                    posy(i) = func_takePoint(seg3_C0,seg3_C1,seg3_C2,seg3_C3,t_dx);
                end
            end
            coef = polyfit(posx,posy,3);
            Valid = true;
            Line_start = seg2_Start;
            Line_end = seg3_end;
            C0 = coef(4);
            C1 = coef(3);
            C2 = coef(2);
            C3 = coef(1);
        end


        %1、3段距离有效
    elseif seg1_valid == true && seg2_valid == false && seg3_valid == true
        if seg3_Start - seg1_Start > single(30)
            seg3_end = seg1_Start;

        else
            seg1_Start = (seg3_Start - seg1_Start)*single(0.5) + seg1_Start;

        end
        t_size = func_step(seg1_end,seg3_end);
        for i = 1:20
            t_dx = seg2_Start + (i - 1)*t_size;
            posx(i) = t_dx;
            if t_dx <= seg1_Start
                posy(i) = func_takePoint(seg1_C0,seg1_C1,seg1_C2,seg1_C3,t_dx);
            else
                posy(i) = func_takePoint(seg3_C0,seg3_C1,seg3_C2,seg3_C3,t_dx);
            end
        end
        coef = polyfit(posx,posy,3);
        Valid = true;
        Line_start = seg1_end;
        Line_end = seg3_end;
        C0 = coef(4);
        C1 = coef(3);
        C2 = coef(2);
        C3 = coef(1);
    end
    %三段有效
else
    if seg1_Start > seg2_Start
        seg2_Start = seg1_Start;
    end
    if seg2_end > seg3_Start
        seg2_end = seg3_Start;
    end
    if seg3_Start >= single(150)
        t_size = func_step(seg1_end,seg2_end);
        for i = 1:20
            t_dx = seg2_Start + (i - 1)*t_size;
            posx(i) = t_dx;
            if t_dx <= seg1_Start
                posy(i) = func_takePoint(seg1_C0,seg1_C1,seg1_C2,seg1_C3,t_dx);
            else
                posy(i) = func_takePoint(seg2_C0,seg2_C1,seg2_C2,seg2_C3,t_dx);

            end
        end
    else
        t_size = func_step(seg1_end,seg3_end);
        for i = 1:20
            t_dx = seg2_Start + (i - 1)*t_size;
            posx(i) = t_dx;
            if t_dx <= seg1_Start
                posy(i) = func_takePoint(seg1_C0,seg1_C1,seg1_C2,seg1_C3,t_dx);
            elseif t_dx > seg1_Start && t_dx <= seg2_end
                posy(i) = func_takePoint(seg2_C0,seg2_C1,seg2_C2,seg2_C3,t_dx);
            else

                posy(i) = func_takePoint(seg3_C0,seg3_C1,seg3_C2,seg3_C3,t_dx);
            end
        end
    end

    coef = polyfit(posx,posy,3);
    Valid = true;
    Line_start = seg1_end;
    Line_end = seg3_end;
    C0 = coef(4);
    C1 = coef(3);
    C2 = coef(2);
    C3 = coef(1);
end
end

%%
%赋值检查函数
function [t_flg,t_start,t_end,t_c0,t_c1,t_c2,t_c3] = func_assign(seg_flg,seg_start,seg_end,seg_c0,seg_c1,seg_c2,seg_c3)
if seg_flg ~= false && seg_start ~= single(0) && seg_end ~= single(0) && seg_c0 ~= single(0) && seg_c1 ~= single(0) && seg_c2 ~= single(0) && seg_c3 ~= single(0)
    t_flg = seg_flg;
    t_start = seg_flg;
    t_end = seg_end;
    t_c0 = seg_c0;
    t_c1 = seg_c1;
    t_c2 = seg_c2;
    t_c3 = seg_c3;
else
    t_flg = false;
    t_start = single(0);
    t_end = single(0);
    t_c0 = single(0);
    t_c1 = single(0);
    t_c2 = single(0);
    t_c3 = single(0);
end
end
% 离散取点函数
function y = func_takePoint(a0,a1,a2,a3,x)
y = a0 + a1*x + a2*x*x + a3*x*x*x;
end

% 取值步长的计算
function size = func_step(t1,t2)
if t2 > single(150)
    t2 = single(150);
end
size = (t2 - t1)* single(0.05);
end

== matlab function TosAeb/ELK_Objsel/LanesSelect/LineInfo_Process1/Line_Info ==
  data: Index output; seg_num input; seg1_valid input; Valid output; seg1_Start input; seg2_valid input; seg2_Start input; seg3_valid input; seg3_Start input
function [Index,Valid] = fcn(seg_num,seg1_valid,seg1_Start,seg2_valid,seg2_Start,seg3_valid,seg3_Start)
Index = uint8(1);
Valid = false;
%确定有效分段数目
if seg_num == uint8(0)

    %只有一段距离有效，输出有效段参数，后续需要增加距离判断拟合
else
    if seg1_valid == true && seg2_valid ==false && seg3_valid == false                                                          %仅第一段有效
        Valid = true;
        Index = uint8(1);
    elseif seg2_valid == true && seg1_valid ==false && seg3_valid == false                                                      %仅第二段有效
        Valid = true;
        Index = uint8(2);
    elseif seg3_valid == true && seg1_valid ==false && seg2_valid == false                                                      %仅第三段有效
        Valid = true;
        Index = uint8(3);
    elseif seg1_valid == true && seg2_valid ==true                                                                              %第一/第二段有效，第三段存在性不考虑
        if seg1_Start > seg2_Start && seg1_Start > single(0)
            Valid =true;
            Index = uint8(1);
        else
            Valid = true;
            Index = uint8(2);
        end
    elseif seg1_valid == true && seg3_valid ==true && seg2_valid == false                                                      %第一/第三段存在，第二段不存在
        if seg1_Start > single(0) && seg3_Start > single(30)
            Valid = true;
            Index = uint8(1);
        else
            Valid = true;
            Index = uint8(3);
        end
    elseif seg1_valid == false && seg3_valid ==true && seg2_valid == true                                                       %第二/第三段存在，第一段不存在
        if seg2_Start < seg3_Start || seg2_Start < single(20)
            Valid = true;
            Index = uint8(2);
        else
            Valid = true;
            Index = uint8(3);
        end
    else
    end
end
end

== matlab function TosAeb/ELK_Objsel/LanesSelect/LineInfo_Process2/Line_Info ==
  data: Index output; seg_num input; seg1_valid input; Valid output; seg1_Start input; seg2_valid input; seg2_Start input; seg3_valid input; seg3_Start input
function [Index,Valid] = fcn(seg_num,seg1_valid,seg1_Start,seg2_valid,seg2_Start,seg3_valid,seg3_Start)
Index = uint8(1);
Valid = false;
%确定有效分段数目
if seg_num == uint8(0)

    %只有一段距离有效，输出有效段参数，后续需要增加距离判断拟合
else
    if seg1_valid == true && seg2_valid ==false && seg3_valid == false                                                          %仅第一段有效
        Valid = true;
        Index = uint8(1);
    elseif seg2_valid == true && seg1_valid ==false && seg3_valid == false                                                      %仅第二段有效
        Valid = true;
        Index = uint8(2);
    elseif seg3_valid == true && seg1_valid ==false && seg2_valid == false                                                      %仅第三段有效
        Valid = true;
        Index = uint8(3);
    elseif seg1_valid == true && seg2_valid ==true                                                                              %第一/第二段有效，第三段存在性不考虑
        if seg1_Start > seg2_Start && seg1_Start > single(0)
            Valid =true;
            Index = uint8(1);
        else
            Valid = true;
            Index = uint8(2);
        end
    elseif seg1_valid == true && seg3_valid ==true && seg2_valid == false                                                      %第一/第三段存在，第二段不存在
        if seg1_Start > single(0) && seg3_Start > single(30)
            Valid = true;
            Index = uint8(1);
        else
            Valid = true;
            Index = uint8(3);
        end
    elseif seg1_valid == false && seg3_valid ==true && seg2_valid == true                                                       %第二/第三段存在，第一段不存在
        if seg2_Start < seg3_Start || seg2_Start < single(20)
            Valid = true;
            Index = uint8(2);
        else
            Valid = true;
            Index = uint8(3);
        end
    else
    end
end
end

== matlab function TosAeb/AEB_Objsel/Lat_Check ==
  data: Lat_ObjID input; ID output; Lat_ObjSortNum input; Lat_TTC input; Num output; Ttc output
function [ID,Num,Ttc] = fcn(Lat_ObjID,Lat_ObjSortNum,Lat_TTC)
t_ID = uint8(zeros(10,1));
t_Num = uint8(zeros(10,1));
t_Ttc=single(zeros(10,1));
for i = 1:10
    if Lat_ObjID(i) == uint8(0)
        t_ID(i) = uint8(0);
        t_Num(i) = uint8(255);
        t_Ttc(i) = single(100);
    else
        t_ID(i) = Lat_ObjID(i);
        t_Num(i) = Lat_ObjSortNum(i);
        t_Ttc(i) = Lat_TTC(i);
    end     
end
ID = t_ID;
Num = t_Num;
Ttc = t_Ttc;
end

== stateflow chart TosAeb/FcwAebJa_Step_sys/ALG_AEBSystem/ALG_AEBController/AEB_FuncCondCalcApp/PedFunctionCondCalc/pedAEBfullSuppAborCond/pedAEBfullAborCondNoStandstillCalc/pedAEBfullObjRelActiveCondCalc/Chart ==
  (default) -> (junction 2)
  (junction 2) -> (junction 3) [order 1] : [targetObjID ~= CNobjInvalidID]
  (junction 2) -> (junction 26) [order 2] : {
      | 	pedAEBfullObjRelAborCondFulfilled = true;
      | }
  (junction 3) -> (junction 16)
  (junction 16) -> (junction 18) [order 1] : [targetObjID == targetObjIDObjCalcK1]
  (junction 16) -> (junction 20) [order 2] : {
      | 	pedAEBfullObjRelAborCondFulfilled = true;
      | }
  (junction 18) -> (junction 5)
  (junction 5) -> (junction 7) [order 1] : [objIsFusionTarget == true]
  (junction 5) -> (junction 11) [order 2]
  (junction 7) -> (junction 9) : {
      | 	pedAEBfullObjRelAborCondFulfilled = false;
      | }
  (junction 11) -> (junction 14) [order 1] : [objLatCollisionProb <= P_pedAEBfulColliisonRiskProbAborThresObjID]
  (junction 11) -> (junction 24) [order 2] : {
      | 	pedAEBfullObjRelAborCondFulfilled = false;
      | }
  (junction 14) -> (junction 22) : {
      | 	pedAEBfullObjRelAborCondFulfilled = true;
      | }
  (junction 20) -> (junction 26)
  (junction 24) -> (junction 20)
  (junction 22) -> (junction 24)
  (junction 9) -> (junction 22)
  (junction 26) -> (junction 32)
  data: targetObjID input; targetObjIDObjCalcK1 input; objIsFusionTarget input; objLatCollisionProb input; pedAEBfullObjRelAborCondFulfilled output boolean; CNobjInvalidID parameter; P_pedAEBfulColliisonRiskProbAborThresObjID input

== stateflow chart TosAeb/FcwAebJa_Step_sys/ALG_AEBSystem/ALG_AEBController/AEB_FuncCondCalcApp/PedFunctionCondCalc/pedAEBfullSuppAborCond/pedAEBfullSuppCondCalc/pedAEBfullSituationRelSuppCondCalc/objLatVyCond/objLatVyCond4FullBrakeCalc ==
  (default) -> (junction 4)
  (junction 4) -> (junction 5) [order 1] : [abs(vy) < P_pedAEBfullVyMaxThres]
  (junction 4) -> (junction 23) [order 2] : {
      | 	objLatVyCond4FullBrake = false;
      | }
  (junction 5) -> (junction 7)
  (junction 7) -> (junction 9) [order 1] : [abs(dy)< P_pedAEBfullDyInnerAreaThres]
  (junction 7) -> (junction 13) [order 2]
  (junction 9) -> (junction 33)
  (junction 33) -> (junction 35) [order 1] : [objFusionType == true]
  (junction 33) -> (junction 37) [order 2] : {
      | 	objLatVyCond4FullBrake = ...
      | (abs(vy) > P_pedAEBfullVyInnerAreaThres4Radar);
      | }
  (junction 35) -> (junction 11) : {
      | 	objLatVyCond4FullBrake = ...
      | (abs(vy) > P_pedAEBfullVyInnerAreaThres4Fusion);
      | }
  (junction 13) -> (junction 15) [order 1] : [abs(dy)< P_pedAEBfullDyMiddleAreaThres]
  (junction 13) -> (junction 50) [order 2]
  (junction 15) -> (junction 42)
  (junction 42) -> (junction 44) [order 1] : [objFusionType == true]
  (junction 42) -> (junction 46) [order 2] : {
      | 	objLatVyCond4FullBrake = ...
      |  (abs(vy) > P_pedAEBfullVyMiddleAreaThres4Radar);
      | }
  (junction 44) -> (junction 17) : {
      | 	objLatVyCond4FullBrake = ...
      | (abs(vy) > P_pedAEBfullVyMiddleAreaThres4Fusion);
      | }
  (junction 50) -> (junction 52) [order 1] : [objFusionType == true]
  (junction 50) -> (junction 19) [order 2] : {
      | 	objLatVyCond4FullBrake =...
      |  (abs(vy) > P_pedAEBfullVyOuterAreaThres4Radar);
      | }
  (junction 52) -> (junction 54) : {
      | 	objLatVyCond4FullBrake = ...
      | (abs(vy) > P_pedAEBfullVyOuterAreaThres4Fusion);
      | }
  (junction 19) -> (junction 23)
  (junction 54) -> (junction 19)
  (junction 46) -> (junction 54)
  (junction 17) -> (junction 46)
  (junction 37) -> (junction 17)
  (junction 11) -> (junction 37)
  (junction 23) -> (junction 26)
  data: objFusionType input; dy input; vy input; objLatVyCond4FullBrake output; P_pedAEBfullVyMaxThres input; P_pedAEBfullDyInnerAreaThres input; P_pedAEBfullDyMiddleAreaThres input; P_pedAEBfullVyInnerAreaThres4Fusion input; P_pedAEBfullVyMiddleAreaThres4Fusion input; P_pedAEBfullVyOuterAreaThres4Fusion input; P_pedAEBfullVyInnerAreaThres4Radar input; P_pedAEBfullVyMiddleAreaThres4Radar input; P_pedAEBfullVyOuterAreaThres4Radar input
